simulink model "TosAeb"  (R2021a)
settings: Solver=FixedStepDiscrete  StartTime=0.0  StopTime=inf  FixedStep=0.01  RelTol=1e-3
undefined symbols (not defined in the file or in its folder): ACCModSts, AccSts1, AEB_ObjectMovingOrientation, AEB_ObjectMovingState, CN_BTCActive, CN_CurrentGearR, CN_CurrentGearR_DHT, CN_PTCActive, CN_VDCActive, CN_VSC_EspOff, CN_VSC_FCW_AEB_Error, CN_VSC_FCW_AEB_NoDispay, CN_VSC_FCW_AEB_PerfmDgdtn, CN_VSC_FCW_AEB_TempUnAvlbl, CN_VSC_VehStandstill, CNaccelAvoidMax, CNaccelAvoidMin, CNbrakePedalPress, CNcomfortStrLeftAccelMax, CNcomfortStrLeftAccelMin, CNcomfortStrRightAccelMax, CNcomfortStrRightAccelMin, CNDrvDoorOpen, CNDrvModeEco, CNDrvModeNorm, CNDrvModeOther, CNDrvModeSport, CNDrvSBRreminder, CNNull, CNobjInvalidID, CNPassDoorOpen, CNPassSBRreminder, CNPEBSAccelNegInf, CNPEBSAccelNull, CNPEBSTimeInf, CNPEBSTimeNull, CNSTOPMAX, CNSTOPNULL, CNsubjWidth, CNSwtOFF, CNSwtON, CNTTCMAX, CNTTCNULL, CNVehConfigAEBCycNone, CNVehConfigAEBNone, CNVehConfigAEBPedNone, CNVehConfigFCWNone, CNVehConfigJANone, CollisionSeverity, FALSE, FCWSnvty, JACycObjCalcInfoDefaultParams, JAPedObjCalcInfoDefaultParams, JAVehObjCalcInfoDefaultParams, latCycObjCalcInfoDefaultParams, latPedObjCalcInfoDefaultParams, longObjCalcInfoDefaultParams, ModSts, movingStatus, ObjectType ... (+1112)
libraries: simulink x3031, AEBSFunctionLib x50, Ta_Library x33, AEBSStateMachineLib x23, Mylibrary x12, simulink_extras x7, ObjSelect_lib x3, dspstat3 x2
(part 89 of 102)

== system TosAeb/AEB_Objsel/TA_Processor/AEB_ObjSelect/TargetInfoCheck/Data_precess/TA_EffectCalculate/Obj_FilterFlgCalculate/Long_ObjFilter/OverlapCalculate/BoudingBoxCalculate_Else/PosnatTTI/PredictHostPositionAtTTR/CalculateHeading/ExtractVelocitiesAtStartOfSegments/ExtractVelocityVectorFirstPoint ==
  Segment : Inport
  FirstPointInSegment : Constant  Value=single(0)
  PositionAndDerivative : MATLAB Function  TreatAsAtomicUnit=on
  PositionAndDerivative1 : MATLAB Function  TreatAsAtomicUnit=on
  SegmentComponents : Subsystem
  Terminator1 : Terminator
  Terminator2 : Terminator
  dx : Outport
  dy : Outport  Port=2
  wires:
  SegmentComponents/6(P1y) -> PositionAndDerivative1/2(P1)
  SegmentComponents/2(P1x) -> PositionAndDerivative/2(P1)
  SegmentComponents/7(P2y) -> PositionAndDerivative1/3(P2)
  SegmentComponents/1(P0x) -> PositionAndDerivative/1(P0)
  SegmentComponents/8(P3y) -> PositionAndDerivative1/4(P3)
  SegmentComponents/4(P3x) -> PositionAndDerivative/4(P3)
  SegmentComponents/3(P2x) -> PositionAndDerivative/3(P2)
  SegmentComponents/5(P0y) -> PositionAndDerivative1/1(P0)
  Segment/1 -> SegmentComponents/1(Segment)
  PositionAndDerivative1/2(dy) -> dy/1
  PositionAndDerivative/1(x) -> Terminator1/1
  FirstPointInSegment/1 -> PositionAndDerivative1/5(tau), PositionAndDerivative/5(tau)
  PositionAndDerivative/2(dx) -> dx/1
  PositionAndDerivative1/1(y) -> Terminator2/1

== system TosAeb/AEB_Objsel/TA_Processor/AEB_ObjSelect/TargetInfoCheck/Data_precess/TA_EffectCalculate/Obj_FilterFlgCalculate/Long_ObjFilter/TTR_Calculate/CalculateTTRAndTTM_else/LongitudinalTTRComputer/CalculateTTI/HostAndObjectMoving/CheckIfSolutionExists/Compare To Zero ==
  u : Inport
  Compare : RelationalOperator  Operator=>  OutDataTypeStr=boolean  RndMeth=Nearest  InputSameDT=on
  Constant : Constant  DisableCoverage=on  Value=0  OutDataTypeStr=Inherit: Inherit via back propagation  PreserveConstantTs=on
  y : Outport
  wires:
  Constant/1 -> Compare/2
  u/1 -> Compare/1
  Compare/1 -> y/1

== system TosAeb/AEB_Objsel/TA_Processor/AEB_ObjSelect/TargetInfoCheck/Data_precess/TA_EffectCalculate/Obj_FilterFlgCalculate/Long_ObjFilter/TTR_Calculate/CalculateTTRAndTTM_else/LongitudinalTTRComputer/CalculateTTI/HostAndObjectMoving/CheckIfSolutionExists/Compare To Zero1 ==
  u : Inport
  Compare : RelationalOperator  Operator=<  OutDataTypeStr=boolean  RndMeth=Nearest  InputSameDT=on
  Constant : Constant  DisableCoverage=on  Value=0  OutDataTypeStr=Inherit: Inherit via back propagation  PreserveConstantTs=on
  y : Outport
  wires:
  Constant/1 -> Compare/2
  u/1 -> Compare/1
  Compare/1 -> y/1

== system TosAeb/AEB_Objsel/TA_Processor/AEB_ObjSelect/TargetInfoCheck/Data_precess/TA_EffectCalculate/Obj_FilterFlgCalculate/Long_ObjFilter/TTR_Calculate/CalculateTTRAndTTM_else/LongitudinalTTRComputer/CalculateTTI/HostAndObjectMoving/CheckIfSolutionExists/LimitedSafeDivide ==
  note: 1e10
  Numerator : Inport
  Denominator : Inport  Port=2
  Add : Sum
  Constant : Constant  Value=P_TA_PrmForTTCalDistanceOffset
  Constant1 : Constant  Value=1e10  OutDataTypeStr=single
  Constant5 : Constant  Value=1e10  OutDataTypeStr=single
  Gain : Gain  Gain=-1  OutDataTypeStr=Inherit: Same as input
  SafeDivide : Subsystem  TreatAsAtomicUnit=on  mask(Safe divide){DataType=single, DataTypeMax=0, DataTypeMin=0}
      mask prompts: DataType "Data type:"
      mask help:
      | This block offers division protected from division by zero.
      |
      | When division by zero is detected, a very large number will be returned with the same sign as the numerator.
      |
      | In the case zero divided by zero, the output has been defined as zero.
      |
      | Both floating-point numbers and integers are supported.
      mask init: same as TosAeb/AEB_Objsel/TA_Processor/PreProcessor/VehiclePreProcessor/CalculateCurvature/SafeDivide
      settings: RTWSystemCode=Inline
      mask Help:
      | This block offers division protected from division by zero.
      | 
      | When division by zero is detected, a very large number will be returned with the same sign as the numerator.
      | 
      | In the case zero divided by zero, the output has been defined as zero.
      | 
      | Both floating-point numbers and integers are supported.
      mask Display:
      | % Author: Jakob Andersson (jande573)
      | 
      | % Display the allowed data type
      | disp(DataType);
      | 
      | % Display the port symbols
      | port_label('input',1,'\bullet','texmode','on');
      | port_label('input',2,'\div','texmode','on');
  Saturation Dynamic : Subsystem  mask(Saturation Dynamic){OutMin=[], OutMax=[], OutDataTypeStr=Inherit: Same as second input, OutputDataTypeScalingMode=Same as second input, OutDataType=fixdt(1, 16), OutScaling=2^-10, LockScale=off, RndMeth=Floor, DoSatur=off}
      mask prompts: OutMin "Simulink:masks:OutputMinimum_MP"; OutMax "Simulink:masks:OutputMaximum_MP"; OutDataTypeStr "Simulink:masks:OutputDataType_MP"; OutputDataTypeScalingMode "Simulink:masks:OutputDataTypeMode_MP"; OutDataType "Simulink:masks:OutputDataTypeegFixdt116Fixdtsingle_MP"; OutScaling "Simulink:masks:OutputScalingSlopeEg29OrSlopeBias_MP"; LockScale "Simulink:masks:LockOutputDataTypeAgainstFxpTools_MP"; RndMeth "Simulink:masks:IntegerRoundingMode_MP"; DoSatur "Simulink:masks:SaturateOnIntegerOverflow_MP"
      mask help: Simulink:masks:SaturationDynamic_MD
      mask init: same as TosAeb/AEB_Objsel/TA_Processor/AEB_ObjSelect/TargetInfoCheck/Data_precess/TA_EffectCalculate/ValideateObj/ObjPos_Calculate/ObjPos_Calculateelse/InPath_Cal/DetermineInPath/LimitedSafeDivide/Saturation Dynamic
      mask Callback OutDataTypeStr: fixptdialog(gcbh)
      mask TypeOptions OutputDataTypeScalingMode: double | single | int8 | uint8 | int16 | uint16 | int32 | uint32 | Specify via dialog | Same as second input
      mask Callback OutputDataTypeScalingMode: fixptdialog(gcbh)
      mask Callback OutDataType: fixptdialog(gcbh)
      mask TypeOptions RndMeth: Simulink:masks:Ceiling | Simulink:masks:Convergent | Simulink:masks:Floor | Simulink:masks:Nearest | Simulink:masks:Round | Simulink:masks:Simplest | Simulink:masks:Zero
      mask Display: plot([0.15 0.85],[.5 .5],[.5 .5],[0.05 0.95],0.15+0.7*[0 .25 .75 1],0.05+0.9*[.25 .25 .75 .75]);
  LimitedQuotient : Outport
  wires:
  Gain/1 -> Saturation Dynamic/3(lo)
  Constant1/1 -> Gain/1
  Constant5/1 -> Saturation Dynamic/1(up)
  SafeDivide/1(Quotient) -> Saturation Dynamic/2(u)
  Denominator/1 -> SafeDivide/2(Denominator)
  Numerator/1 -> SafeDivide/1(Numerator)
  Saturation Dynamic/1(y) -> Add/1
  Add/1 -> LimitedQuotient/1
  Constant/1 -> Add/2

== system TosAeb/AEB_Objsel/TA_Processor/AEB_ObjSelect/TargetInfoCheck/Data_precess/TA_EffectCalculate/Obj_FilterFlgCalculate/Long_ObjFilter/TTR_Calculate/CalculateTTRAndTTM_else/LongitudinalTTRComputer/CalculateTTI/HostAndObjectMoving/CheckIfSolutionExists/SizeChecker ==
  Signal : Inport  OutDataTypeStr=DataTypeIn
      settings: AttributesFormatString=DT=%<OutDataTypeStr>
  Abs : Abs  SaturateOnIntegerOverflow=on
  LimitSource : Constant  Value=Limit  OutDataTypeStr=Inherit: Inherit via back propagation
      settings: AttributesFormatString=DT=%<OutDataTypeStr>
  Relational Operator : RelationalOperator  Operator=>  OutDataTypeStr=boolean  RndMeth=Nearest  InputSameDT=on
      settings: AttributesFormatString=InputSameDT=%<InputSameDT>
  TestOk : Outport  OutDataTypeStr=boolean
      settings: AttributesFormatString=DT=%<OutDataTypeStr>
  wires:
  Relational Operator/1 -> TestOk/1  "TestOk"
  Signal/1 -> Abs/1  "Signal"
  LimitSource/1 -> Relational Operator/2  "LimitSource"
  Abs/1 -> Relational Operator/1  "AbsoluteSignal"

== system TosAeb/AEB_Objsel/TA_Processor/AEB_ObjSelect/TargetInfoCheck/Data_precess/TA_EffectCalculate/Obj_FilterFlgCalculate/Long_ObjFilter/TTR_Calculate/CalculateTTRAndTTM_else/LongitudinalTTRComputer/CalculateTTI/HostAndObjectMoving/CheckIfSolutionExists1/Compare To Zero ==
  u : Inport
  Compare : RelationalOperator  Operator=>  OutDataTypeStr=boolean  RndMeth=Nearest  InputSameDT=on
  Constant : Constant  DisableCoverage=on  Value=0  OutDataTypeStr=Inherit: Inherit via back propagation  PreserveConstantTs=on
  y : Outport
  wires:
  Constant/1 -> Compare/2
  u/1 -> Compare/1
  Compare/1 -> y/1

== system TosAeb/AEB_Objsel/TA_Processor/AEB_ObjSelect/TargetInfoCheck/Data_precess/TA_EffectCalculate/Obj_FilterFlgCalculate/Long_ObjFilter/TTR_Calculate/CalculateTTRAndTTM_else/LongitudinalTTRComputer/CalculateTTI/HostAndObjectMoving/CheckIfSolutionExists1/Compare To Zero1 ==
  u : Inport
  Compare : RelationalOperator  Operator=<  OutDataTypeStr=boolean  RndMeth=Nearest  InputSameDT=on
  Constant : Constant  DisableCoverage=on  Value=0  OutDataTypeStr=Inherit: Inherit via back propagation  PreserveConstantTs=on
  y : Outport
  wires:
  Constant/1 -> Compare/2
  u/1 -> Compare/1
  Compare/1 -> y/1

== system TosAeb/AEB_Objsel/TA_Processor/AEB_ObjSelect/TargetInfoCheck/Data_precess/TA_EffectCalculate/Obj_FilterFlgCalculate/Long_ObjFilter/TTR_Calculate/CalculateTTRAndTTM_else/LongitudinalTTRComputer/CalculateTTI/HostAndObjectMoving/CheckIfSolutionExists1/LimitedSafeDivide ==
  note: 1e10
  Numerator : Inport
  Denominator : Inport  Port=2
  Add : Sum  Inputs=+-
  Constant : Constant  Value=P_TA_PrmForTTCalDistanceOffset
  Constant1 : Constant  Value=1e10
  Constant5 : Constant  Value=1e10
  Gain : Gain  Gain=-1  OutDataTypeStr=Inherit: Same as input
  SafeDivide : Subsystem  TreatAsAtomicUnit=on  mask(Safe divide){DataType=single, DataTypeMax=0, DataTypeMin=0}
      mask prompts: DataType "Data type:"
      mask help:
      | This block offers division protected from division by zero.
      |
      | When division by zero is detected, a very large number will be returned with the same sign as the numerator.
      |
      | In the case zero divided by zero, the output has been defined as zero.
      |
      | Both floating-point numbers and integers are supported.
      mask init: same as TosAeb/AEB_Objsel/TA_Processor/PreProcessor/VehiclePreProcessor/CalculateCurvature/SafeDivide
      settings: RTWSystemCode=Inline
      mask Help:
      | This block offers division protected from division by zero.
      | 
      | When division by zero is detected, a very large number will be returned with the same sign as the numerator.
      | 
      | In the case zero divided by zero, the output has been defined as zero.
      | 
      | Both floating-point numbers and integers are supported.
      mask Display:
      | % Author: Jakob Andersson (jande573)
      | 
      | % Display the allowed data type
      | disp(DataType);
      | 
      | % Display the port symbols
      | port_label('input',1,'\bullet','texmode','on');
      | port_label('input',2,'\div','texmode','on');
  Saturation Dynamic : Subsystem  mask(Saturation Dynamic){OutMin=[], OutMax=[], OutDataTypeStr=Inherit: Same as second input, OutputDataTypeScalingMode=Same as second input, OutDataType=fixdt(1, 16), OutScaling=2^-10, LockScale=off, RndMeth=Floor, DoSatur=off}
      mask prompts: OutMin "Simulink:masks:OutputMinimum_MP"; OutMax "Simulink:masks:OutputMaximum_MP"; OutDataTypeStr "Simulink:masks:OutputDataType_MP"; OutputDataTypeScalingMode "Simulink:masks:OutputDataTypeMode_MP"; OutDataType "Simulink:masks:OutputDataTypeegFixdt116Fixdtsingle_MP"; OutScaling "Simulink:masks:OutputScalingSlopeEg29OrSlopeBias_MP"; LockScale "Simulink:masks:LockOutputDataTypeAgainstFxpTools_MP"; RndMeth "Simulink:masks:IntegerRoundingMode_MP"; DoSatur "Simulink:masks:SaturateOnIntegerOverflow_MP"
      mask help: Simulink:masks:SaturationDynamic_MD
      mask init: same as TosAeb/AEB_Objsel/TA_Processor/AEB_ObjSelect/TargetInfoCheck/Data_precess/TA_EffectCalculate/ValideateObj/ObjPos_Calculate/ObjPos_Calculateelse/InPath_Cal/DetermineInPath/LimitedSafeDivide/Saturation Dynamic
      mask Callback OutDataTypeStr: fixptdialog(gcbh)
      mask TypeOptions OutputDataTypeScalingMode: double | single | int8 | uint8 | int16 | uint16 | int32 | uint32 | Specify via dialog | Same as second input
      mask Callback OutputDataTypeScalingMode: fixptdialog(gcbh)
      mask Callback OutDataType: fixptdialog(gcbh)
      mask TypeOptions RndMeth: Simulink:masks:Ceiling | Simulink:masks:Convergent | Simulink:masks:Floor | Simulink:masks:Nearest | Simulink:masks:Round | Simulink:masks:Simplest | Simulink:masks:Zero
      mask Display: plot([0.15 0.85],[.5 .5],[.5 .5],[0.05 0.95],0.15+0.7*[0 .25 .75 1],0.05+0.9*[.25 .25 .75 .75]);
  LimitedQuotient : Outport
  wires:
  Gain/1 -> Saturation Dynamic/3(lo)
  Constant1/1 -> Gain/1
  Constant5/1 -> Saturation Dynamic/1(up)
  Saturation Dynamic/1(y) -> Add/1
  SafeDivide/1(Quotient) -> Saturation Dynamic/2(u)
  Denominator/1 -> SafeDivide/2(Denominator)
  Numerator/1 -> SafeDivide/1(Numerator)
  Add/1 -> LimitedQuotient/1
  Constant/1 -> Add/2

== system TosAeb/AEB_Objsel/TA_Processor/AEB_ObjSelect/TargetInfoCheck/Data_precess/TA_EffectCalculate/Obj_FilterFlgCalculate/Long_ObjFilter/TTR_Calculate/CalculateTTRAndTTM_else/LongitudinalTTRComputer/CalculateTTI/HostAndObjectMoving/CheckIfSolutionExists1/SizeChecker ==
  Signal : Inport  OutDataTypeStr=DataTypeIn
      settings: AttributesFormatString=DT=%<OutDataTypeStr>
  Abs : Abs  SaturateOnIntegerOverflow=on
  LimitSource : Constant  Value=Limit  OutDataTypeStr=Inherit: Inherit via back propagation
      settings: AttributesFormatString=DT=%<OutDataTypeStr>
  Relational Operator : RelationalOperator  Operator=>  OutDataTypeStr=boolean  RndMeth=Nearest  InputSameDT=on
      settings: AttributesFormatString=InputSameDT=%<InputSameDT>
  TestOk : Outport  OutDataTypeStr=boolean
      settings: AttributesFormatString=DT=%<OutDataTypeStr>
  wires:
  Relational Operator/1 -> TestOk/1  "TestOk"
  Signal/1 -> Abs/1  "Signal"
  LimitSource/1 -> Relational Operator/2  "LimitSource"
  Abs/1 -> Relational Operator/1  "AbsoluteSignal"

== system TosAeb/AEB_Objsel/TA_Processor/AEB_ObjSelect/TargetInfoCheck/Data_precess/TA_EffectCalculate/Obj_FilterFlgCalculate/Long_ObjFilter/TTR_Calculate/CalculateTTRAndTTM_else/LongitudinalTTRComputer/CalculateTTI/HostAndObjectMoving/TimeToIntersectionComputer/Compare To Zero ==
  u : Inport
  Compare : RelationalOperator  Operator=<  OutDataTypeStr=boolean  RndMeth=Nearest  InputSameDT=on
  Constant : Constant  DisableCoverage=on  Value=0  OutDataTypeStr=Inherit: Inherit via back propagation  PreserveConstantTs=on
  y : Outport
  wires:
  Constant/1 -> Compare/2
  u/1 -> Compare/1
  Compare/1 -> y/1

== system TosAeb/AEB_Objsel/TA_Processor/AEB_ObjSelect/TargetInfoCheck/Data_precess/TA_EffectCalculate/Obj_FilterFlgCalculate/Long_ObjFilter/TTR_Calculate/CalculateTTRAndTTM_else/LongitudinalTTRComputer/CalculateTTI/HostAndObjectMoving/TimeToIntersectionComputer/SolutionChooser ==
  note: If ARel is negative, choose the largest solution.
      | Otherwise, choose the smallest solution.
  Solutions : Inport
  ARel : Inport  Port=2  OutDataTypeStr=single
      settings: AttributesFormatString=DT=%<OutDataTypeStr>
  Compare To Zero1 : Subsystem  mask(Compare To Zero){relop=<, OutDataTypeStr=boolean, ZeroCross=on}
      mask prompts: relop "Simulink:masks:Operator_MP"; OutDataTypeStr "Simulink:masks:OutputDataType_MP"; ZeroCross "Simulink:masks:EnableZerocrossingDetection_MP"
      mask help: Simulink:masks:CompareToZero_MD
      mask init: same as TosAeb/AEB_Objsel/TA_Processor/AEB_ObjSelect/TargetInfoCheck/Data_precess/TA_EffectCalculate/Obj_FilterFlgCalculate/Long_ObjFilter/TTR_Calculate/CalculateTTRAndTTM_else/ModifyTTRAtSameSpeedAndAcceleration/Compare To Zero
      mask TypeOptions relop: == | ~= | < | <= | >= | >
      mask TypeOptions OutDataTypeStr: Simulink:masks:uint8 | Simulink:masks:boolean
      mask Callback ZeroCross: sl('zeroCrossEnable', gcbh);
      mask Display: disp([relop,' 0'])
  Selector : Selector  InputPortWidth=2  IndexOptions=Index vector (dialog)  Indices=1  OutputSizes=1
  Selector1 : Selector  InputPortWidth=2  IndexOptions=Index vector (dialog)  Indices=2  OutputSizes=1
  Switch3 : Switch  Criteria=u2 ~= 0  InputSameDT=on
      settings: AttributesFormatString=InputSameDT=%<InputSameDT>
  Solution : Outport
  wires:
  Selector1/1 -> Switch3/3
  Solutions/1 -> [Solutions], Selector/1
  Selector/1 -> Switch3/1
  Switch3/1 -> Solution/1  "Solution"
  ARel/1 -> Compare To Zero1/1(u)
  Compare To Zero1/1(y) -> Switch3/2  "NegativeARel"
  [Solutions] -> Selector1/1

== system TosAeb/AEB_Objsel/TA_Processor/AEB_ObjSelect/TargetInfoCheck/Data_precess/TA_EffectCalculate/Obj_FilterFlgCalculate/Long_ObjFilter/TTR_Calculate/CalculateTTRAndTTM_else/LongitudinalTTRComputer/CalculateTTI/HostAndObjectMoving/TimeToIntersectionComputer1/Compare To Zero ==
  u : Inport
  Compare : RelationalOperator  Operator=<  OutDataTypeStr=boolean  RndMeth=Nearest  InputSameDT=on
  Constant : Constant  DisableCoverage=on  Value=0  OutDataTypeStr=Inherit: Inherit via back propagation  PreserveConstantTs=on
  y : Outport
  wires:
  Compare/1 -> y/1
  u/1 -> Compare/1
  Constant/1 -> Compare/2

== system TosAeb/AEB_Objsel/TA_Processor/AEB_ObjSelect/TargetInfoCheck/Data_precess/TA_EffectCalculate/Obj_FilterFlgCalculate/Long_ObjFilter/TTR_Calculate/CalculateTTRAndTTM_else/LongitudinalTTRComputer/CalculateTTI/HostAndObjectMoving/TimeToIntersectionComputer1/SolutionChooser ==
  note: If ARel is negative, choose the largest solution.
      | Otherwise, choose the smallest solution.
  Solutions : Inport
  ARel : Inport  Port=2  OutDataTypeStr=single
      settings: AttributesFormatString=DT=%<OutDataTypeStr>
  Compare To Zero1 : Subsystem  mask(Compare To Zero){relop=<, OutDataTypeStr=boolean, ZeroCross=on}
      mask prompts: relop "Simulink:masks:Operator_MP"; OutDataTypeStr "Simulink:masks:OutputDataType_MP"; ZeroCross "Simulink:masks:EnableZerocrossingDetection_MP"
      mask help: Simulink:masks:CompareToZero_MD
      mask init: same as TosAeb/AEB_Objsel/TA_Processor/AEB_ObjSelect/TargetInfoCheck/Data_precess/TA_EffectCalculate/Obj_FilterFlgCalculate/Long_ObjFilter/TTR_Calculate/CalculateTTRAndTTM_else/ModifyTTRAtSameSpeedAndAcceleration/Compare To Zero
      mask TypeOptions relop: == | ~= | < | <= | >= | >
      mask TypeOptions OutDataTypeStr: Simulink:masks:uint8 | Simulink:masks:boolean
      mask Callback ZeroCross: sl('zeroCrossEnable', gcbh);
      mask Display: disp([relop,' 0'])
  Selector : Selector  InputPortWidth=2  IndexOptions=Index vector (dialog)  Indices=1  OutputSizes=1
  Selector1 : Selector  InputPortWidth=2  IndexOptions=Index vector (dialog)  Indices=2  OutputSizes=1
  Switch3 : Switch  Criteria=u2 ~= 0  InputSameDT=on
      settings: AttributesFormatString=InputSameDT=%<InputSameDT>
  Solution : Outport
  wires:
  [Solutions] -> Selector1/1
  Compare To Zero1/1(y) -> Switch3/2  "NegativeARel"
  ARel/1 -> Compare To Zero1/1(u)
  Switch3/1 -> Solution/1  "Solution"
  Selector/1 -> Switch3/1
  Solutions/1 -> Selector/1, [Solutions]
  Selector1/1 -> Switch3/3

== system TosAeb/AEB_Objsel/TA_Processor/AEB_ObjSelect/TargetInfoCheck/Data_precess/TA_EffectCalculate/Obj_FilterFlgCalculate/Long_ObjFilter/TTR_Calculate/CalculateTTRAndTTM_else/LongitudinalTTRComputer/CalculateTTI/HostAndObjectMoving/TimeToIntersectionComputer1/Subsystem ==
  ax : Inport
  Abs : Abs
  Add : Sum  Inputs=+-
  Add1 : Sum
  Compare To Constant : simulink/Logic and Bit Operations/Compare To Constant  relop=>=  const=0.1  OutDataTypeStr=boolean
  Constant : Constant  Value=0.001  OutDataTypeStr=single
  Product : Product
  Switch : Switch  Criteria=u2 > Threshold
  Unit Delay : UnitDelay  SampleTime=-1  HasFrameUpgradeWarning=on
  Out1 : Outport
  wires:
  Add1/1 -> Switch/1
  Constant/1 -> Product/1
  Product/1 -> Add1/2
  Compare To Constant/1 -> Switch/2
  Abs/1 -> Compare To Constant/1
  Unit Delay/1 -> Add/2, Add1/1
  Switch/1 -> Unit Delay/1, Out1/1
  Add/1 -> Abs/1, Product/2
  ax/1 -> Add/1, Switch/3

== system TosAeb/AEB_Objsel/TA_Processor/AEB_ObjSelect/TargetInfoCheck/Data_precess/TA_EffectCalculate/Obj_FilterFlgCalculate/Long_ObjFilter/TTR_Calculate/CalculateTTRAndTTM_else/LongitudinalTTRComputer/CalculateTTI/StationaryHost/CheckIfSolutionExists/Compare To Zero ==
  u : Inport
  Compare : RelationalOperator  Operator=>  OutDataTypeStr=boolean  RndMeth=Nearest  InputSameDT=on
  Constant : Constant  DisableCoverage=on  Value=0  OutDataTypeStr=Inherit: Inherit via back propagation  PreserveConstantTs=on
  y : Outport
  wires:
  Constant/1 -> Compare/2
  u/1 -> Compare/1
  Compare/1 -> y/1

== system TosAeb/AEB_Objsel/TA_Processor/AEB_ObjSelect/TargetInfoCheck/Data_precess/TA_EffectCalculate/Obj_FilterFlgCalculate/Long_ObjFilter/TTR_Calculate/CalculateTTRAndTTM_else/LongitudinalTTRComputer/CalculateTTI/StationaryHost/CheckIfSolutionExists/Compare To Zero1 ==
  u : Inport
  Compare : RelationalOperator  Operator=<  OutDataTypeStr=boolean  RndMeth=Nearest  InputSameDT=on
  Constant : Constant  DisableCoverage=on  Value=0  OutDataTypeStr=Inherit: Inherit via back propagation  PreserveConstantTs=on
  y : Outport
  wires:
  Constant/1 -> Compare/2
  u/1 -> Compare/1
  Compare/1 -> y/1

== system TosAeb/AEB_Objsel/TA_Processor/AEB_ObjSelect/TargetInfoCheck/Data_precess/TA_EffectCalculate/Obj_FilterFlgCalculate/Long_ObjFilter/TTR_Calculate/CalculateTTRAndTTM_else/LongitudinalTTRComputer/CalculateTTI/StationaryHost/CheckIfSolutionExists/LimitedSafeDivide ==
  note: 1e10
  Numerator : Inport
  Denominator : Inport  Port=2
  Add : Sum
  Constant : Constant  Value=P_TA_PrmForTTCalDistanceOffset
  Constant1 : Constant  Value=1e10
  Constant5 : Constant  Value=1e10
  Gain : Gain  Gain=-1  OutDataTypeStr=Inherit: Same as input
  SafeDivide : Subsystem  TreatAsAtomicUnit=on  mask(Safe divide){DataType=single, DataTypeMax=0, DataTypeMin=0}
      mask prompts: DataType "Data type:"
      mask help:
      | This block offers division protected from division by zero.
      |
      | When division by zero is detected, a very large number will be returned with the same sign as the numerator.
      |
      | In the case zero divided by zero, the output has been defined as zero.
      |
      | Both floating-point numbers and integers are supported.
      mask init: same as TosAeb/AEB_Objsel/TA_Processor/PreProcessor/VehiclePreProcessor/CalculateCurvature/SafeDivide
      settings: RTWSystemCode=Inline
      mask Help:
      | This block offers division protected from division by zero.
      | 
      | When division by zero is detected, a very large number will be returned with the same sign as the numerator.
      | 
      | In the case zero divided by zero, the output has been defined as zero.
      | 
      | Both floating-point numbers and integers are supported.
      mask Display:
      | % Author: Jakob Andersson (jande573)
      | 
      | % Display the allowed data type
      | disp(DataType);
      | 
      | % Display the port symbols
      | port_label('input',1,'\bullet','texmode','on');
      | port_label('input',2,'\div','texmode','on');
  Saturation Dynamic : Subsystem  mask(Saturation Dynamic){OutMin=[], OutMax=[], OutDataTypeStr=Inherit: Same as second input, OutputDataTypeScalingMode=Same as second input, OutDataType=fixdt(1, 16), OutScaling=2^-10, LockScale=off, RndMeth=Floor, DoSatur=off}
      mask prompts: OutMin "Simulink:masks:OutputMinimum_MP"; OutMax "Simulink:masks:OutputMaximum_MP"; OutDataTypeStr "Simulink:masks:OutputDataType_MP"; OutputDataTypeScalingMode "Simulink:masks:OutputDataTypeMode_MP"; OutDataType "Simulink:masks:OutputDataTypeegFixdt116Fixdtsingle_MP"; OutScaling "Simulink:masks:OutputScalingSlopeEg29OrSlopeBias_MP"; LockScale "Simulink:masks:LockOutputDataTypeAgainstFxpTools_MP"; RndMeth "Simulink:masks:IntegerRoundingMode_MP"; DoSatur "Simulink:masks:SaturateOnIntegerOverflow_MP"
      mask help: Simulink:masks:SaturationDynamic_MD
      mask init: same as TosAeb/AEB_Objsel/TA_Processor/AEB_ObjSelect/TargetInfoCheck/Data_precess/TA_EffectCalculate/ValideateObj/ObjPos_Calculate/ObjPos_Calculateelse/InPath_Cal/DetermineInPath/LimitedSafeDivide/Saturation Dynamic
      mask Callback OutDataTypeStr: fixptdialog(gcbh)
      mask TypeOptions OutputDataTypeScalingMode: double | single | int8 | uint8 | int16 | uint16 | int32 | uint32 | Specify via dialog | Same as second input
      mask Callback OutputDataTypeScalingMode: fixptdialog(gcbh)
      mask Callback OutDataType: fixptdialog(gcbh)
      mask TypeOptions RndMeth: Simulink:masks:Ceiling | Simulink:masks:Convergent | Simulink:masks:Floor | Simulink:masks:Nearest | Simulink:masks:Round | Simulink:masks:Simplest | Simulink:masks:Zero
      mask Display: plot([0.15 0.85],[.5 .5],[.5 .5],[0.05 0.95],0.15+0.7*[0 .25 .75 1],0.05+0.9*[.25 .25 .75 .75]);
  LimitedQuotient : Outport
  wires:
  Gain/1 -> Saturation Dynamic/3(lo)
  Constant1/1 -> Gain/1
  Constant5/1 -> Saturation Dynamic/1(up)
  SafeDivide/1(Quotient) -> Saturation Dynamic/2(u)
  Denominator/1 -> SafeDivide/2(Denominator)
  Numerator/1 -> SafeDivide/1(Numerator)
  Saturation Dynamic/1(y) -> Add/1
  Constant/1 -> Add/2
  Add/1 -> LimitedQuotient/1

== system TosAeb/AEB_Objsel/TA_Processor/AEB_ObjSelect/TargetInfoCheck/Data_precess/TA_EffectCalculate/Obj_FilterFlgCalculate/Long_ObjFilter/TTR_Calculate/CalculateTTRAndTTM_else/LongitudinalTTRComputer/CalculateTTI/StationaryHost/CheckIfSolutionExists/SizeChecker ==
  Signal : Inport  OutDataTypeStr=DataTypeIn
      settings: AttributesFormatString=DT=%<OutDataTypeStr>
  Abs : Abs  SaturateOnIntegerOverflow=on
  LimitSource : Constant  Value=Limit  OutDataTypeStr=Inherit: Inherit via back propagation
      settings: AttributesFormatString=DT=%<OutDataTypeStr>
  Relational Operator : RelationalOperator  Operator=>  OutDataTypeStr=boolean  RndMeth=Nearest  InputSameDT=on
      settings: AttributesFormatString=InputSameDT=%<InputSameDT>
  TestOk : Outport  OutDataTypeStr=boolean
      settings: AttributesFormatString=DT=%<OutDataTypeStr>
  wires:
  Relational Operator/1 -> TestOk/1  "TestOk"
  Signal/1 -> Abs/1  "Signal"
  LimitSource/1 -> Relational Operator/2  "LimitSource"
  Abs/1 -> Relational Operator/1  "AbsoluteSignal"

== system TosAeb/AEB_Objsel/TA_Processor/AEB_ObjSelect/TargetInfoCheck/Data_precess/TA_EffectCalculate/Obj_FilterFlgCalculate/Long_ObjFilter/TTR_Calculate/CalculateTTRAndTTM_else/LongitudinalTTRComputer/CalculateTTI/StationaryHost/CheckIfSolutionExists1/Compare To Zero ==
  u : Inport
  Compare : RelationalOperator  Operator=>  OutDataTypeStr=boolean  RndMeth=Nearest  InputSameDT=on
  Constant : Constant  DisableCoverage=on  Value=0  OutDataTypeStr=Inherit: Inherit via back propagation  PreserveConstantTs=on
  y : Outport
  wires:
  Constant/1 -> Compare/2
  u/1 -> Compare/1
  Compare/1 -> y/1

== system TosAeb/AEB_Objsel/TA_Processor/AEB_ObjSelect/TargetInfoCheck/Data_precess/TA_EffectCalculate/Obj_FilterFlgCalculate/Long_ObjFilter/TTR_Calculate/CalculateTTRAndTTM_else/LongitudinalTTRComputer/CalculateTTI/StationaryHost/CheckIfSolutionExists1/Compare To Zero1 ==
  u : Inport
  Compare : RelationalOperator  Operator=<  OutDataTypeStr=boolean  RndMeth=Nearest  InputSameDT=on
  Constant : Constant  DisableCoverage=on  Value=0  OutDataTypeStr=Inherit: Inherit via back propagation  PreserveConstantTs=on
  y : Outport
  wires:
  Constant/1 -> Compare/2
  u/1 -> Compare/1
  Compare/1 -> y/1

== system TosAeb/AEB_Objsel/TA_Processor/AEB_ObjSelect/TargetInfoCheck/Data_precess/TA_EffectCalculate/Obj_FilterFlgCalculate/Long_ObjFilter/TTR_Calculate/CalculateTTRAndTTM_else/LongitudinalTTRComputer/CalculateTTI/StationaryHost/CheckIfSolutionExists1/LimitedSafeDivide ==
  note: 1e10
  Numerator : Inport
  Denominator : Inport  Port=2
  Add : Sum  Inputs=+-
  Constant : Constant  Value=P_TA_PrmForTTCalDistanceOffset
  Constant1 : Constant  Value=1e10
  Constant5 : Constant  Value=1e10
  Gain : Gain  Gain=-1  OutDataTypeStr=Inherit: Same as input
  SafeDivide : Subsystem  TreatAsAtomicUnit=on  mask(Safe divide){DataType=single, DataTypeMax=0, DataTypeMin=0}
      mask prompts: DataType "Data type:"
      mask help:
      | This block offers division protected from division by zero.
      |
      | When division by zero is detected, a very large number will be returned with the same sign as the numerator.
      |
      | In the case zero divided by zero, the output has been defined as zero.
      |
      | Both floating-point numbers and integers are supported.
      mask init: same as TosAeb/AEB_Objsel/TA_Processor/PreProcessor/VehiclePreProcessor/CalculateCurvature/SafeDivide
      settings: RTWSystemCode=Inline
      mask Help:
      | This block offers division protected from division by zero.
      | 
      | When division by zero is detected, a very large number will be returned with the same sign as the numerator.
      | 
      | In the case zero divided by zero, the output has been defined as zero.
      | 
      | Both floating-point numbers and integers are supported.
      mask Display:
      | % Author: Jakob Andersson (jande573)
      | 
      | % Display the allowed data type
      | disp(DataType);
      | 
      | % Display the port symbols
      | port_label('input',1,'\bullet','texmode','on');
      | port_label('input',2,'\div','texmode','on');
  Saturation Dynamic : Subsystem  mask(Saturation Dynamic){OutMin=[], OutMax=[], OutDataTypeStr=Inherit: Same as second input, OutputDataTypeScalingMode=Same as second input, OutDataType=fixdt(1, 16), OutScaling=2^-10, LockScale=off, RndMeth=Floor, DoSatur=off}
      mask prompts: OutMin "Simulink:masks:OutputMinimum_MP"; OutMax "Simulink:masks:OutputMaximum_MP"; OutDataTypeStr "Simulink:masks:OutputDataType_MP"; OutputDataTypeScalingMode "Simulink:masks:OutputDataTypeMode_MP"; OutDataType "Simulink:masks:OutputDataTypeegFixdt116Fixdtsingle_MP"; OutScaling "Simulink:masks:OutputScalingSlopeEg29OrSlopeBias_MP"; LockScale "Simulink:masks:LockOutputDataTypeAgainstFxpTools_MP"; RndMeth "Simulink:masks:IntegerRoundingMode_MP"; DoSatur "Simulink:masks:SaturateOnIntegerOverflow_MP"
      mask help: Simulink:masks:SaturationDynamic_MD
      mask init: same as TosAeb/AEB_Objsel/TA_Processor/AEB_ObjSelect/TargetInfoCheck/Data_precess/TA_EffectCalculate/ValideateObj/ObjPos_Calculate/ObjPos_Calculateelse/InPath_Cal/DetermineInPath/LimitedSafeDivide/Saturation Dynamic
      mask Callback OutDataTypeStr: fixptdialog(gcbh)
      mask TypeOptions OutputDataTypeScalingMode: double | single | int8 | uint8 | int16 | uint16 | int32 | uint32 | Specify via dialog | Same as second input
      mask Callback OutputDataTypeScalingMode: fixptdialog(gcbh)
      mask Callback OutDataType: fixptdialog(gcbh)
      mask TypeOptions RndMeth: Simulink:masks:Ceiling | Simulink:masks:Convergent | Simulink:masks:Floor | Simulink:masks:Nearest | Simulink:masks:Round | Simulink:masks:Simplest | Simulink:masks:Zero
      mask Display: plot([0.15 0.85],[.5 .5],[.5 .5],[0.05 0.95],0.15+0.7*[0 .25 .75 1],0.05+0.9*[.25 .25 .75 .75]);
  LimitedQuotient : Outport
  wires:
  Gain/1 -> Saturation Dynamic/3(lo)
  Constant1/1 -> Gain/1
  Constant5/1 -> Saturation Dynamic/1(up)
  SafeDivide/1(Quotient) -> Saturation Dynamic/2(u)
  Denominator/1 -> SafeDivide/2(Denominator)
  Numerator/1 -> SafeDivide/1(Numerator)
  Constant/1 -> Add/2
  Saturation Dynamic/1(y) -> Add/1
  Add/1 -> LimitedQuotient/1

== system TosAeb/AEB_Objsel/TA_Processor/AEB_ObjSelect/TargetInfoCheck/Data_precess/TA_EffectCalculate/Obj_FilterFlgCalculate/Long_ObjFilter/TTR_Calculate/CalculateTTRAndTTM_else/LongitudinalTTRComputer/CalculateTTI/StationaryHost/CheckIfSolutionExists1/SizeChecker ==
  Signal : Inport  OutDataTypeStr=DataTypeIn
      settings: AttributesFormatString=DT=%<OutDataTypeStr>
  Abs : Abs  SaturateOnIntegerOverflow=on
  LimitSource : Constant  Value=Limit  OutDataTypeStr=Inherit: Inherit via back propagation
      settings: AttributesFormatString=DT=%<OutDataTypeStr>
  Relational Operator : RelationalOperator  Operator=>  OutDataTypeStr=boolean  RndMeth=Nearest  InputSameDT=on
      settings: AttributesFormatString=InputSameDT=%<InputSameDT>
  TestOk : Outport  OutDataTypeStr=boolean
      settings: AttributesFormatString=DT=%<OutDataTypeStr>
  wires:
  Relational Operator/1 -> TestOk/1  "TestOk"
  Signal/1 -> Abs/1  "Signal"
  LimitSource/1 -> Relational Operator/2  "LimitSource"
  Abs/1 -> Relational Operator/1  "AbsoluteSignal"

== system TosAeb/AEB_Objsel/TA_Processor/AEB_ObjSelect/TargetInfoCheck/Data_precess/TA_EffectCalculate/Obj_FilterFlgCalculate/Long_ObjFilter/TTR_Calculate/CalculateTTRAndTTM_else/LongitudinalTTRComputer/CalculateTTI/StationaryHost/TimeToIntersectionComputer/Compare To Zero ==
  u : Inport
  Compare : RelationalOperator  Operator=<  OutDataTypeStr=boolean  RndMeth=Nearest  InputSameDT=on
  Constant : Constant  DisableCoverage=on  Value=0  OutDataTypeStr=Inherit: Inherit via back propagation  PreserveConstantTs=on
  y : Outport
  wires:
  Constant/1 -> Compare/2
  u/1 -> Compare/1
  Compare/1 -> y/1

== system TosAeb/AEB_Objsel/TA_Processor/AEB_ObjSelect/TargetInfoCheck/Data_precess/TA_EffectCalculate/Obj_FilterFlgCalculate/Long_ObjFilter/TTR_Calculate/CalculateTTRAndTTM_else/LongitudinalTTRComputer/CalculateTTI/StationaryHost/TimeToIntersectionComputer/SolutionChooser ==
  note: If ARel is negative, choose the largest solution.
      | Otherwise, choose the smallest solution.
  Solutions : Inport
  ARel : Inport  Port=2  OutDataTypeStr=single
      settings: AttributesFormatString=DT=%<OutDataTypeStr>
  Compare To Zero1 : Subsystem  mask(Compare To Zero){relop=<, OutDataTypeStr=boolean, ZeroCross=on}
      mask prompts: relop "Simulink:masks:Operator_MP"; OutDataTypeStr "Simulink:masks:OutputDataType_MP"; ZeroCross "Simulink:masks:EnableZerocrossingDetection_MP"
      mask help: Simulink:masks:CompareToZero_MD
      mask init: same as TosAeb/AEB_Objsel/TA_Processor/AEB_ObjSelect/TargetInfoCheck/Data_precess/TA_EffectCalculate/Obj_FilterFlgCalculate/Long_ObjFilter/TTR_Calculate/CalculateTTRAndTTM_else/ModifyTTRAtSameSpeedAndAcceleration/Compare To Zero
      mask TypeOptions relop: == | ~= | < | <= | >= | >
      mask TypeOptions OutDataTypeStr: Simulink:masks:uint8 | Simulink:masks:boolean
      mask Callback ZeroCross: sl('zeroCrossEnable', gcbh);
      mask Display: disp([relop,' 0'])
  Selector : Selector  InputPortWidth=2  IndexOptions=Index vector (dialog)  Indices=1  OutputSizes=1
  Selector1 : Selector  InputPortWidth=2  IndexOptions=Index vector (dialog)  Indices=2  OutputSizes=1
  Switch3 : Switch  Criteria=u2 ~= 0  InputSameDT=on
      settings: AttributesFormatString=InputSameDT=%<InputSameDT>
  Solution : Outport
  wires:
  Selector1/1 -> Switch3/3
  Solutions/1 -> [Solutions], Selector/1
  Selector/1 -> Switch3/1
  Switch3/1 -> Solution/1  "Solution"
  ARel/1 -> Compare To Zero1/1(u)
  Compare To Zero1/1(y) -> Switch3/2  "NegativeARel"
  [Solutions] -> Selector1/1

== system TosAeb/AEB_Objsel/TA_Processor/AEB_ObjSelect/TargetInfoCheck/Data_precess/TA_EffectCalculate/Obj_FilterFlgCalculate/Long_ObjFilter/TTR_Calculate/CalculateTTRAndTTM_else/LongitudinalTTRComputer/CalculateTTI/StationaryHost/TimeToIntersectionComputer1/Compare To Zero ==
  u : Inport
  Compare : RelationalOperator  Operator=<  OutDataTypeStr=boolean  RndMeth=Nearest  InputSameDT=on
  Constant : Constant  DisableCoverage=on  Value=0  OutDataTypeStr=Inherit: Inherit via back propagation  PreserveConstantTs=on
  y : Outport
  wires:
  Compare/1 -> y/1
  u/1 -> Compare/1
  Constant/1 -> Compare/2

== system TosAeb/AEB_Objsel/TA_Processor/AEB_ObjSelect/TargetInfoCheck/Data_precess/TA_EffectCalculate/Obj_FilterFlgCalculate/Long_ObjFilter/TTR_Calculate/CalculateTTRAndTTM_else/LongitudinalTTRComputer/CalculateTTI/StationaryHost/TimeToIntersectionComputer1/SolutionChooser ==
  note: If ARel is negative, choose the largest solution.
      | Otherwise, choose the smallest solution.
  Solutions : Inport
  ARel : Inport  Port=2  OutDataTypeStr=single
      settings: AttributesFormatString=DT=%<OutDataTypeStr>
  Compare To Zero1 : Subsystem  mask(Compare To Zero){relop=<, OutDataTypeStr=boolean, ZeroCross=on}
      mask prompts: relop "Simulink:masks:Operator_MP"; OutDataTypeStr "Simulink:masks:OutputDataType_MP"; ZeroCross "Simulink:masks:EnableZerocrossingDetection_MP"
      mask help: Simulink:masks:CompareToZero_MD
      mask init: same as TosAeb/AEB_Objsel/TA_Processor/AEB_ObjSelect/TargetInfoCheck/Data_precess/TA_EffectCalculate/Obj_FilterFlgCalculate/Long_ObjFilter/TTR_Calculate/CalculateTTRAndTTM_else/ModifyTTRAtSameSpeedAndAcceleration/Compare To Zero
      mask TypeOptions relop: == | ~= | < | <= | >= | >
      mask TypeOptions OutDataTypeStr: Simulink:masks:uint8 | Simulink:masks:boolean
      mask Callback ZeroCross: sl('zeroCrossEnable', gcbh);
      mask Display: disp([relop,' 0'])
  Selector : Selector  InputPortWidth=2  IndexOptions=Index vector (dialog)  Indices=1  OutputSizes=1
  Selector1 : Selector  InputPortWidth=2  IndexOptions=Index vector (dialog)  Indices=2  OutputSizes=1
  Switch3 : Switch  Criteria=u2 ~= 0  InputSameDT=on
      settings: AttributesFormatString=InputSameDT=%<InputSameDT>
  Solution : Outport
  wires:
  [Solutions] -> Selector1/1
  Compare To Zero1/1(y) -> Switch3/2  "NegativeARel"
  ARel/1 -> Compare To Zero1/1(u)
  Switch3/1 -> Solution/1  "Solution"
  Selector/1 -> Switch3/1
  Solutions/1 -> Selector/1, [Solutions]
  Selector1/1 -> Switch3/3

== system TosAeb/AEB_Objsel/TA_Processor/AEB_ObjSelect/TargetInfoCheck/Data_precess/TA_EffectCalculate/Obj_FilterFlgCalculate/Long_ObjFilter/TTR_Calculate/CalculateTTRAndTTM_else/LongitudinalTTRComputer/CalculateTTI/StationaryObject/CheckIfSolutionExists/Compare To Zero ==
  u : Inport
  Compare : RelationalOperator  Operator=>  OutDataTypeStr=boolean  RndMeth=Nearest  InputSameDT=on
  Constant : Constant  DisableCoverage=on  Value=0  OutDataTypeStr=Inherit: Inherit via back propagation  PreserveConstantTs=on
  y : Outport
  wires:
  Constant/1 -> Compare/2
  u/1 -> Compare/1
  Compare/1 -> y/1

== system TosAeb/AEB_Objsel/TA_Processor/AEB_ObjSelect/TargetInfoCheck/Data_precess/TA_EffectCalculate/Obj_FilterFlgCalculate/Long_ObjFilter/TTR_Calculate/CalculateTTRAndTTM_else/LongitudinalTTRComputer/CalculateTTI/StationaryObject/CheckIfSolutionExists/Compare To Zero1 ==
  u : Inport
  Compare : RelationalOperator  Operator=<  OutDataTypeStr=boolean  RndMeth=Nearest  InputSameDT=on
  Constant : Constant  DisableCoverage=on  Value=0  OutDataTypeStr=Inherit: Inherit via back propagation  PreserveConstantTs=on
  y : Outport
  wires:
  Constant/1 -> Compare/2
  u/1 -> Compare/1
  Compare/1 -> y/1

== system TosAeb/AEB_Objsel/TA_Processor/AEB_ObjSelect/TargetInfoCheck/Data_precess/TA_EffectCalculate/Obj_FilterFlgCalculate/Long_ObjFilter/TTR_Calculate/CalculateTTRAndTTM_else/LongitudinalTTRComputer/CalculateTTI/StationaryObject/CheckIfSolutionExists/LimitedSafeDivide ==
  note: 1e10
  Numerator : Inport
  Denominator : Inport  Port=2
  Add : Sum
  Constant : Constant  Value=P_TA_PrmForTTCalDistanceOffset
  Constant1 : Constant  Value=1e10
  Constant5 : Constant  Value=1e10
  Gain : Gain  Gain=-1  OutDataTypeStr=Inherit: Same as input
  SafeDivide : Subsystem  TreatAsAtomicUnit=on  mask(Safe divide){DataType=single, DataTypeMax=0, DataTypeMin=0}
      mask prompts: DataType "Data type:"
      mask help:
      | This block offers division protected from division by zero.
      |
      | When division by zero is detected, a very large number will be returned with the same sign as the numerator.
      |
      | In the case zero divided by zero, the output has been defined as zero.
      |
      | Both floating-point numbers and integers are supported.
      mask init: same as TosAeb/AEB_Objsel/TA_Processor/PreProcessor/VehiclePreProcessor/CalculateCurvature/SafeDivide
      settings: RTWSystemCode=Inline
      mask Help:
      | This block offers division protected from division by zero.
      | 
      | When division by zero is detected, a very large number will be returned with the same sign as the numerator.
      | 
      | In the case zero divided by zero, the output has been defined as zero.
      | 
      | Both floating-point numbers and integers are supported.
      mask Display:
      | % Author: Jakob Andersson (jande573)
      | 
      | % Display the allowed data type
      | disp(DataType);
      | 
      | % Display the port symbols
      | port_label('input',1,'\bullet','texmode','on');
      | port_label('input',2,'\div','texmode','on');
  Saturation Dynamic : Subsystem  mask(Saturation Dynamic){OutMin=[], OutMax=[], OutDataTypeStr=Inherit: Same as second input, OutputDataTypeScalingMode=Same as second input, OutDataType=fixdt(1, 16), OutScaling=2^-10, LockScale=off, RndMeth=Floor, DoSatur=off}
      mask prompts: OutMin "Simulink:masks:OutputMinimum_MP"; OutMax "Simulink:masks:OutputMaximum_MP"; OutDataTypeStr "Simulink:masks:OutputDataType_MP"; OutputDataTypeScalingMode "Simulink:masks:OutputDataTypeMode_MP"; OutDataType "Simulink:masks:OutputDataTypeegFixdt116Fixdtsingle_MP"; OutScaling "Simulink:masks:OutputScalingSlopeEg29OrSlopeBias_MP"; LockScale "Simulink:masks:LockOutputDataTypeAgainstFxpTools_MP"; RndMeth "Simulink:masks:IntegerRoundingMode_MP"; DoSatur "Simulink:masks:SaturateOnIntegerOverflow_MP"
      mask help: Simulink:masks:SaturationDynamic_MD
      mask init: same as TosAeb/AEB_Objsel/TA_Processor/AEB_ObjSelect/TargetInfoCheck/Data_precess/TA_EffectCalculate/ValideateObj/ObjPos_Calculate/ObjPos_Calculateelse/InPath_Cal/DetermineInPath/LimitedSafeDivide/Saturation Dynamic
      mask Callback OutDataTypeStr: fixptdialog(gcbh)
      mask TypeOptions OutputDataTypeScalingMode: double | single | int8 | uint8 | int16 | uint16 | int32 | uint32 | Specify via dialog | Same as second input
      mask Callback OutputDataTypeScalingMode: fixptdialog(gcbh)
      mask Callback OutDataType: fixptdialog(gcbh)
      mask TypeOptions RndMeth: Simulink:masks:Ceiling | Simulink:masks:Convergent | Simulink:masks:Floor | Simulink:masks:Nearest | Simulink:masks:Round | Simulink:masks:Simplest | Simulink:masks:Zero
      mask Display: plot([0.15 0.85],[.5 .5],[.5 .5],[0.05 0.95],0.15+0.7*[0 .25 .75 1],0.05+0.9*[.25 .25 .75 .75]);
  LimitedQuotient : Outport
  wires:
  Gain/1 -> Saturation Dynamic/3(lo)
  Constant1/1 -> Gain/1
  Constant5/1 -> Saturation Dynamic/1(up)
  SafeDivide/1(Quotient) -> Saturation Dynamic/2(u)
  Denominator/1 -> SafeDivide/2(Denominator)
  Numerator/1 -> SafeDivide/1(Numerator)
  Constant/1 -> Add/2
  Saturation Dynamic/1(y) -> Add/1
  Add/1 -> LimitedQuotient/1

== system TosAeb/AEB_Objsel/TA_Processor/AEB_ObjSelect/TargetInfoCheck/Data_precess/TA_EffectCalculate/Obj_FilterFlgCalculate/Long_ObjFilter/TTR_Calculate/CalculateTTRAndTTM_else/LongitudinalTTRComputer/CalculateTTI/StationaryObject/CheckIfSolutionExists/SizeChecker ==
  Signal : Inport  OutDataTypeStr=DataTypeIn
      settings: AttributesFormatString=DT=%<OutDataTypeStr>
  Abs : Abs  SaturateOnIntegerOverflow=on
  LimitSource : Constant  Value=Limit  OutDataTypeStr=Inherit: Inherit via back propagation
      settings: AttributesFormatString=DT=%<OutDataTypeStr>
  Relational Operator : RelationalOperator  Operator=>  OutDataTypeStr=boolean  RndMeth=Nearest  InputSameDT=on
      settings: AttributesFormatString=InputSameDT=%<InputSameDT>
  TestOk : Outport  OutDataTypeStr=boolean
      settings: AttributesFormatString=DT=%<OutDataTypeStr>
  wires:
  Relational Operator/1 -> TestOk/1  "TestOk"
  Signal/1 -> Abs/1  "Signal"
  LimitSource/1 -> Relational Operator/2  "LimitSource"
  Abs/1 -> Relational Operator/1  "AbsoluteSignal"

== system TosAeb/AEB_Objsel/TA_Processor/AEB_ObjSelect/TargetInfoCheck/Data_precess/TA_EffectCalculate/Obj_FilterFlgCalculate/Long_ObjFilter/TTR_Calculate/CalculateTTRAndTTM_else/LongitudinalTTRComputer/CalculateTTI/StationaryObject/CheckIfSolutionExists1/Compare To Zero ==
  u : Inport
  Compare : RelationalOperator  Operator=>  OutDataTypeStr=boolean  RndMeth=Nearest  InputSameDT=on
  Constant : Constant  DisableCoverage=on  Value=0  OutDataTypeStr=Inherit: Inherit via back propagation  PreserveConstantTs=on
  y : Outport
  wires:
  Constant/1 -> Compare/2
  u/1 -> Compare/1
  Compare/1 -> y/1

== system TosAeb/AEB_Objsel/TA_Processor/AEB_ObjSelect/TargetInfoCheck/Data_precess/TA_EffectCalculate/Obj_FilterFlgCalculate/Long_ObjFilter/TTR_Calculate/CalculateTTRAndTTM_else/LongitudinalTTRComputer/CalculateTTI/StationaryObject/CheckIfSolutionExists1/Compare To Zero1 ==
  u : Inport
  Compare : RelationalOperator  Operator=<  OutDataTypeStr=boolean  RndMeth=Nearest  InputSameDT=on
  Constant : Constant  DisableCoverage=on  Value=0  OutDataTypeStr=Inherit: Inherit via back propagation  PreserveConstantTs=on
  y : Outport
  wires:
  Constant/1 -> Compare/2
  u/1 -> Compare/1
  Compare/1 -> y/1

== system TosAeb/AEB_Objsel/TA_Processor/AEB_ObjSelect/TargetInfoCheck/Data_precess/TA_EffectCalculate/Obj_FilterFlgCalculate/Long_ObjFilter/TTR_Calculate/CalculateTTRAndTTM_else/LongitudinalTTRComputer/CalculateTTI/StationaryObject/CheckIfSolutionExists1/LimitedSafeDivide ==
  Numerator : Inport
  Denominator : Inport  Port=2
  Add : Sum  Inputs=+-
  Constant : Constant  Value=P_TA_PrmForTTCalDistanceOffset
  Constant1 : Constant  Value=1e10  OutDataTypeStr=single
  Constant5 : Constant  Value=1e10  OutDataTypeStr=single
  Gain : Gain  Gain=-1  OutDataTypeStr=Inherit: Same as input
  SafeDivide : Subsystem  TreatAsAtomicUnit=on  mask(Safe divide){DataType=single, DataTypeMax=0, DataTypeMin=0}
      mask prompts: DataType "Data type:"
      mask help:
      | This block offers division protected from division by zero.
      |
      | When division by zero is detected, a very large number will be returned with the same sign as the numerator.
      |
      | In the case zero divided by zero, the output has been defined as zero.
      |
      | Both floating-point numbers and integers are supported.
      mask init: same as TosAeb/AEB_Objsel/TA_Processor/PreProcessor/VehiclePreProcessor/CalculateCurvature/SafeDivide
      settings: RTWSystemCode=Inline
      mask Help:
      | This block offers division protected from division by zero.
      | 
      | When division by zero is detected, a very large number will be returned with the same sign as the numerator.
      | 
      | In the case zero divided by zero, the output has been defined as zero.
      | 
      | Both floating-point numbers and integers are supported.
      mask Display:
      | % Author: Jakob Andersson (jande573)
      | 
      | % Display the allowed data type
      | disp(DataType);
      | 
      | % Display the port symbols
      | port_label('input',1,'\bullet','texmode','on');
      | port_label('input',2,'\div','texmode','on');
  Saturation Dynamic : Subsystem  mask(Saturation Dynamic){OutMin=[], OutMax=[], OutDataTypeStr=Inherit: Same as second input, OutputDataTypeScalingMode=Same as second input, OutDataType=fixdt(1, 16), OutScaling=2^-10, LockScale=off, RndMeth=Floor, DoSatur=off}
      mask prompts: OutMin "Simulink:masks:OutputMinimum_MP"; OutMax "Simulink:masks:OutputMaximum_MP"; OutDataTypeStr "Simulink:masks:OutputDataType_MP"; OutputDataTypeScalingMode "Simulink:masks:OutputDataTypeMode_MP"; OutDataType "Simulink:masks:OutputDataTypeegFixdt116Fixdtsingle_MP"; OutScaling "Simulink:masks:OutputScalingSlopeEg29OrSlopeBias_MP"; LockScale "Simulink:masks:LockOutputDataTypeAgainstFxpTools_MP"; RndMeth "Simulink:masks:IntegerRoundingMode_MP"; DoSatur "Simulink:masks:SaturateOnIntegerOverflow_MP"
      mask help: Simulink:masks:SaturationDynamic_MD
      mask init: same as TosAeb/AEB_Objsel/TA_Processor/AEB_ObjSelect/TargetInfoCheck/Data_precess/TA_EffectCalculate/ValideateObj/ObjPos_Calculate/ObjPos_Calculateelse/InPath_Cal/DetermineInPath/LimitedSafeDivide/Saturation Dynamic
      mask Callback OutDataTypeStr: fixptdialog(gcbh)
      mask TypeOptions OutputDataTypeScalingMode: double | single | int8 | uint8 | int16 | uint16 | int32 | uint32 | Specify via dialog | Same as second input
      mask Callback OutputDataTypeScalingMode: fixptdialog(gcbh)
      mask Callback OutDataType: fixptdialog(gcbh)
      mask TypeOptions RndMeth: Simulink:masks:Ceiling | Simulink:masks:Convergent | Simulink:masks:Floor | Simulink:masks:Nearest | Simulink:masks:Round | Simulink:masks:Simplest | Simulink:masks:Zero
      mask Display: plot([0.15 0.85],[.5 .5],[.5 .5],[0.05 0.95],0.15+0.7*[0 .25 .75 1],0.05+0.9*[.25 .25 .75 .75]);
  LimitedQuotient : Outport
  wires:
  Gain/1 -> Saturation Dynamic/3(lo)
  Constant1/1 -> Gain/1
  Constant5/1 -> Saturation Dynamic/1(up)
  SafeDivide/1(Quotient) -> Saturation Dynamic/2(u)
  Denominator/1 -> SafeDivide/2(Denominator)
  Numerator/1 -> SafeDivide/1(Numerator)
  Constant/1 -> Add/2
  Saturation Dynamic/1(y) -> Add/1
  Add/1 -> LimitedQuotient/1
